simulink model "dark_pfb_core10"  (R2012b)
settings: Solver=ode45  StartTime=0.0  StopTime=10.0  RelTol=1e-3
undefined symbols (not defined in the file or in its folder): pfb_coeff_gen_calc
libraries: xbsIndex_r4 x32067, casper_library_misc x268, casper_library_delays x22, xps_library x1
(part 1 of 300)
settings Model: Creator=kids  LastModifiedBy=kids

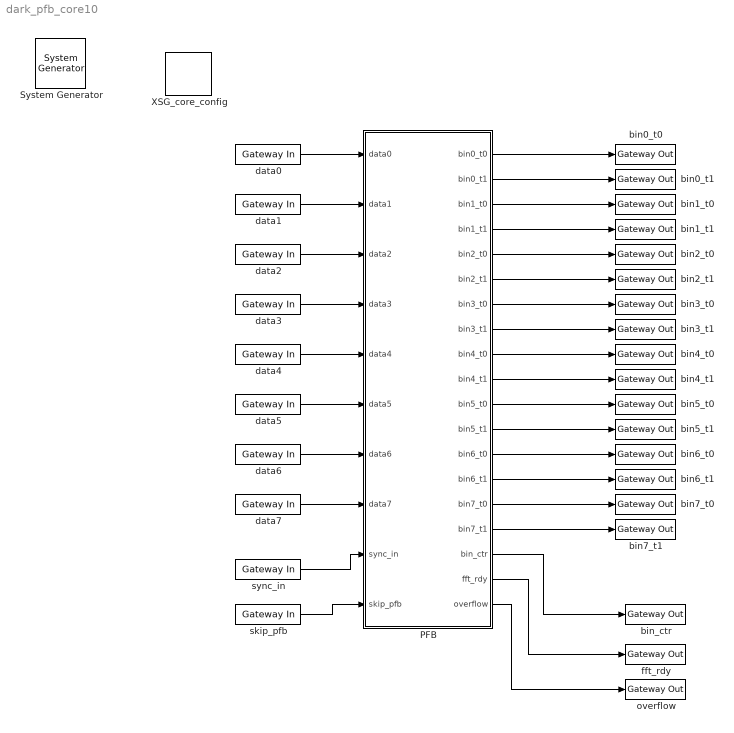
== system dark_pfb_core10 ==
  " System Generator" : xbsIndex_r4/ System Generator  Tag=genX  xilinxfamily=virtex6  part=xc6vsx475t  speed=-1  package=ff1759  synthesis_tool=XST  clock_wrapper=Clock Enables  directory=./dark_pfb_core10/sysgen  proj_type=Project Navigator  Synth_file=XST Defaults  Impl_file=ISE Defaults  testbench=off  simulink_period=1  sysclk_period=4  dcm_input_clock_period=100  incr_netlist=off  trim_vbits=Everywhere in SubSystem  dbl_ovrd=According to Block Masks  core_generation=According to Block Masks  run_coregen=off  deprecated_control=off  eval_field=0  block_type=sysgen
  PFB : Subsystem
  XSG_core_config : xps_library/XSG_core_config  Tag=xps:xsg  hw_sys=ROACH2:sx475t  clk_src=sys_clk  ROACH_clk_src=sys_clk  ROACH2_clk_src=sys_clk  MKDIG_clk_src=sys_clk  clk_rate=250  sample_period=1  synthesis_tool=XST
  bin0_t0 : xbsIndex_r4/Gateway Out  inherit_from_input=off  hdl_port=on  timing_constraint=None  locs_specified=off  LOCs={}  UseAsDAC=off  DACChannel='1'  block_type=gatewayout
  bin0_t1 : xbsIndex_r4/Gateway Out  inherit_from_input=off  hdl_port=on  timing_constraint=None  locs_specified=off  LOCs={}  UseAsDAC=off  DACChannel='1'  block_type=gatewayout
  bin1_t0 : xbsIndex_r4/Gateway Out  inherit_from_input=off  hdl_port=on  timing_constraint=None  locs_specified=off  LOCs={}  UseAsDAC=off  DACChannel='1'  block_type=gatewayout
  bin1_t1 : xbsIndex_r4/Gateway Out  inherit_from_input=off  hdl_port=on  timing_constraint=None  locs_specified=off  LOCs={}  UseAsDAC=off  DACChannel='1'  block_type=gatewayout
  bin2_t0 : xbsIndex_r4/Gateway Out  inherit_from_input=off  hdl_port=on  timing_constraint=None  locs_specified=off  LOCs={}  UseAsDAC=off  DACChannel='1'  block_type=gatewayout
  bin2_t1 : xbsIndex_r4/Gateway Out  inherit_from_input=off  hdl_port=on  timing_constraint=None  locs_specified=off  LOCs={}  UseAsDAC=off  DACChannel='1'  block_type=gatewayout
  bin3_t0 : xbsIndex_r4/Gateway Out  inherit_from_input=off  hdl_port=on  timing_constraint=None  locs_specified=off  LOCs={}  UseAsDAC=off  DACChannel='1'  block_type=gatewayout
  bin3_t1 : xbsIndex_r4/Gateway Out  inherit_from_input=off  hdl_port=on  timing_constraint=None  locs_specified=off  LOCs={}  UseAsDAC=off  DACChannel='1'  block_type=gatewayout
  bin4_t0 : xbsIndex_r4/Gateway Out  inherit_from_input=off  hdl_port=on  timing_constraint=None  locs_specified=off  LOCs={}  UseAsDAC=off  DACChannel='1'  block_type=gatewayout
  bin4_t1 : xbsIndex_r4/Gateway Out  inherit_from_input=off  hdl_port=on  timing_constraint=None  locs_specified=off  LOCs={}  UseAsDAC=off  DACChannel='1'  block_type=gatewayout
  bin5_t0 : xbsIndex_r4/Gateway Out  inherit_from_input=off  hdl_port=on  timing_constraint=None  locs_specified=off  LOCs={}  UseAsDAC=off  DACChannel='1'  block_type=gatewayout
  bin5_t1 : xbsIndex_r4/Gateway Out  inherit_from_input=off  hdl_port=on  timing_constraint=None  locs_specified=off  LOCs={}  UseAsDAC=off  DACChannel='1'  block_type=gatewayout
  bin6_t0 : xbsIndex_r4/Gateway Out  inherit_from_input=off  hdl_port=on  timing_constraint=None  locs_specified=off  LOCs={}  UseAsDAC=off  DACChannel='1'  block_type=gatewayout
  bin6_t1 : xbsIndex_r4/Gateway Out  inherit_from_input=off  hdl_port=on  timing_constraint=None  locs_specified=off  LOCs={}  UseAsDAC=off  DACChannel='1'  block_type=gatewayout
  bin7_t0 : xbsIndex_r4/Gateway Out  inherit_from_input=off  hdl_port=on  timing_constraint=None  locs_specified=off  LOCs={}  UseAsDAC=off  DACChannel='1'  block_type=gatewayout
  bin7_t1 : xbsIndex_r4/Gateway Out  inherit_from_input=off  hdl_port=on  timing_constraint=None  locs_specified=off  LOCs={}  UseAsDAC=off  DACChannel='1'  block_type=gatewayout
  bin_ctr : xbsIndex_r4/Gateway Out  inherit_from_input=off  hdl_port=on  timing_constraint=None  locs_specified=off  LOCs={}  UseAsDAC=off  DACChannel='1'  block_type=gatewayout
  data{0..7} (8 alike) : xbsIndex_r4/Gateway In  gui_display_data_type=Fixed-point  arith_type=Unsigned  n_bits=24  bin_pt=0  preci_type=Single  exp_width=8  frac_width=24  quantization="Round  (unbiased: +/- Inf)"  overflow=Saturate  period=1  dbl_ovrd=off  timing_constraint=None  locs_specified=off  LOCs={}  UseAsADC=off  ADCChannel='1'  inherit_from_input=off  hdl_port=on  block_type=gatewayin
  fft_rdy : xbsIndex_r4/Gateway Out  inherit_from_input=off  hdl_port=on  timing_constraint=None  locs_specified=off  LOCs={}  UseAsDAC=off  DACChannel='1'  block_type=gatewayout
  overflow : xbsIndex_r4/Gateway Out  inherit_from_input=off  hdl_port=on  timing_constraint=None  locs_specified=off  LOCs={}  UseAsDAC=off  DACChannel='1'  block_type=gatewayout
  skip_pfb : xbsIndex_r4/Gateway In  gui_display_data_type=Boolean  arith_type=Boolean  n_bits=16  bin_pt=14  preci_type=Single  exp_width=8  frac_width=24  quantization="Round  (unbiased: +/- Inf)"  overflow=Saturate  period=1  dbl_ovrd=off  timing_constraint=None  locs_specified=off  LOCs={}  UseAsADC=off  ADCChannel='1'  inherit_from_input=off  hdl_port=on  block_type=gatewayin
  sync_in : xbsIndex_r4/Gateway In  gui_display_data_type=Boolean  arith_type=Boolean  n_bits=16  bin_pt=14  preci_type=Single  exp_width=8  frac_width=24  quantization="Round  (unbiased: +/- Inf)"  overflow=Saturate  period=1  dbl_ovrd=off  timing_constraint=None  locs_specified=off  LOCs={}  UseAsADC=off  ADCChannel='1'  inherit_from_input=off  hdl_port=on  block_type=gatewayin
  wires:
  PFB/18(fft_rdy) -> fft_rdy/1
  data0/1 -> PFB/1(data0)
  data1/1 -> PFB/2(data1)
  data2/1 -> PFB/3(data2)
  data3/1 -> PFB/4(data3)
  data4/1 -> PFB/5(data4)
  data5/1 -> PFB/6(data5)
  data6/1 -> PFB/7(data6)
  data7/1 -> PFB/8(data7)
  sync_in/1 -> PFB/9(sync_in)
  PFB/19(overflow) -> overflow/1
  PFB/17(bin_ctr) -> bin_ctr/1
  skip_pfb/1 -> PFB/10(skip_pfb)
  PFB/16(bin7_t1) -> bin7_t1/1
  PFB/15(bin7_t0) -> bin7_t0/1
  PFB/14(bin6_t1) -> bin6_t1/1
  PFB/13(bin6_t0) -> bin6_t0/1
  PFB/12(bin5_t1) -> bin5_t1/1
  PFB/11(bin5_t0) -> bin5_t0/1
  PFB/10(bin4_t1) -> bin4_t1/1
  PFB/9(bin4_t0) -> bin4_t0/1
  PFB/8(bin3_t1) -> bin3_t1/1
  PFB/7(bin3_t0) -> bin3_t0/1
  PFB/6(bin2_t1) -> bin2_t1/1
  PFB/5(bin2_t0) -> bin2_t0/1
  PFB/4(bin1_t1) -> bin1_t1/1
  PFB/3(bin1_t0) -> bin1_t0/1
  PFB/2(bin0_t1) -> bin0_t1/1
  PFB/1(bin0_t0) -> bin0_t0/1

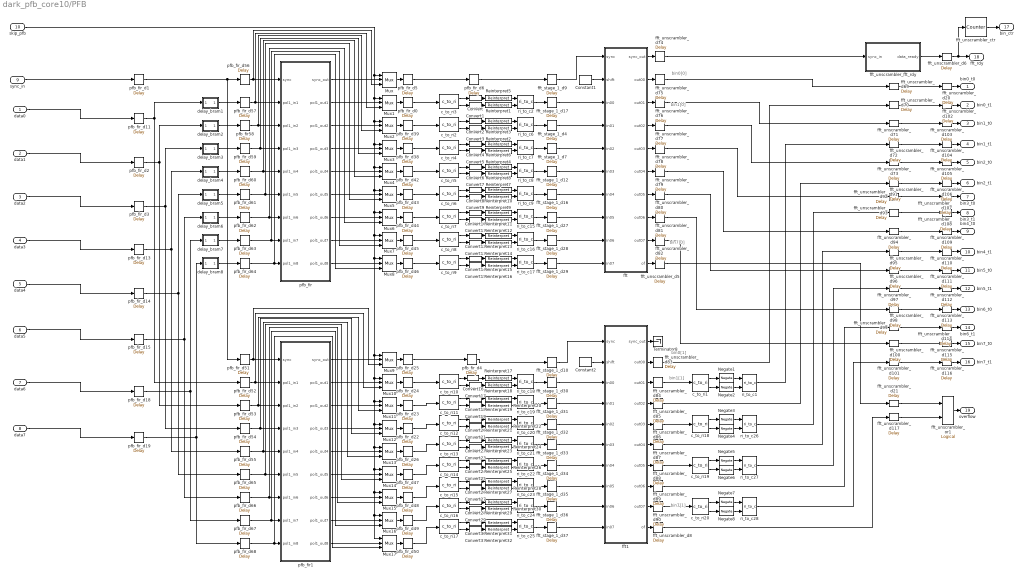
== system dark_pfb_core10/PFB ==
  note: bin7[1]
  note: bin7[0]
  note: bin1[1]
  note: bin1[0]
  note: bin0[1]
  note: bin0[0]
  data0 : Inport
  data1 : Inport  Port=2
  data2 : Inport  Port=3
  data3 : Inport  Port=4
  data4 : Inport  Port=5
  data5 : Inport  Port=6
  data6 : Inport  Port=7
  data7 : Inport  Port=8
  sync_in : Inport  Port=9
  skip_pfb : Inport  Port=10
  Constant1 : xbsIndex_r4/Constant  const=2^5-1  gui_display_data_type=Fixed-point  arith_type=Unsigned  n_bits=32  bin_pt=0  preci_type=Single  exp_width=8  frac_width=24  explicit_period=on  period=1  equ=P=C  opselect=C  inp2=PCIN>>17  opr=+  inp1=P  carry=CIN  dbl_ovrd=off  block_type=constant
  Constant2 : xbsIndex_r4/Constant  const=2^5-1  gui_display_data_type=Fixed-point  arith_type=Unsigned  n_bits=32  bin_pt=0  preci_type=Single  exp_width=8  frac_width=24  explicit_period=on  period=1  equ=P=C  opselect=C  inp2=PCIN>>17  opr=+  inp1=P  carry=CIN  dbl_ovrd=off  block_type=constant
  Convert{,1..31} (32 alike) : xbsIndex_r4/Convert  gui_display_data_type=Fixed-point  arith_type=Unsigned  n_bits=18  bin_pt=17  float_type=Single  exp_bits=8  fraction_bits=24  quantization="Round  (unbiased: +/- Inf)"  overflow=Wrap  en=off  latency=2  dbl_ovrd=off  pipeline=on  block_type=convert
      settings: block_version=11.4
  Mux{,1..17} (18 alike) : xbsIndex_r4/Mux  inputs=2  en=off  latency=0  precision=Full  arith_type=Unsigned  n_bits=16  bin_pt=14  quantization=Truncate  overflow=Wrap  dbl_ovrd=off  block_type=mux
  Negate{1..8} (8 alike) : xbsIndex_r4/Negate  precision=User Defined  arith_type="Signed  (2's comp)"  n_bits=18  bin_pt=17  quantization=Truncate  overflow=Wrap  en=off  latency=0  dbl_ovrd=off  block_type=negate
      settings: block_version=11.4
  Reinterpret{1..32} (32 alike) : xbsIndex_r4/Reinterpret  force_arith_type=off  arith_type="Signed  (2's comp)"  force_bin_pt=on  bin_pt=0  block_type=reinterpret
      settings: infoedit="Changes signal type without altering the binary representation.   You can changed the signal between signed and unsigned, and relocate the binary point.<br><br>Hardware notes: In hardware this block costs nothing.<br><br>Example:  Suppose the input is 6 bits wide, signed, with 2 fractional bits, and the output is forced to unsigned with 0 fractional bits.  Then an input of -2.0 (1110.00 in binary 2's complement) becomes an output of 56 (111000 in binary)."
  Terminator8 : Terminator
  c_to_ri{1,18..20} (4 alike) : casper_library_misc/c_to_ri  n_bits=18  bin_pt=17
  c_to_ri{2..17} (16 alike) : casper_library_misc/c_to_ri  n_bits=12  bin_pt=11
      settings: AttributesFormatString=12_11 r/i
  delay_bram1 : Subsystem  AncestorBlock=casper_library_delays/delay_bram  mask(delay_bram){DelayLen=128, bram_latency=2, use_dsp48=on, async=off}
      mask prompts: DelayLen "Delay By:"; bram_latency "BRAM Latency"; use_dsp48 "Use DSP48"; async "asynchronous operation"
      mask help: A delay block that uses BRAM for its storage.
      mask init:
      | delay_bram_init(gcb, ...
      |     'DelayLen', DelayLen, ...
      |     'bram_latency', bram_latency, ...
      |     'use_dsp48', use_dsp48, ...
      |     'async', async);
      mask Help: eval('xlWeb(''http://casper.berkeley.edu/wiki/Delay_bram'')')
      mask Display:
      | str=sprintf('z^{-%d}',DelayLen);
      | color('black');disp(str,'texmode','on');
  delay_bram2 : Subsystem  AncestorBlock=casper_library_delays/delay_bram  mask(delay_bram){DelayLen=128, bram_latency=2, use_dsp48=on, async=off}
      mask prompts: DelayLen "Delay By:"; bram_latency "BRAM Latency"; use_dsp48 "Use DSP48"; async "asynchronous operation"
      mask help: A delay block that uses BRAM for its storage.
      mask init:
      | delay_bram_init(gcb, ...
      |     'DelayLen', DelayLen, ...
      |     'bram_latency', bram_latency, ...
      |     'use_dsp48', use_dsp48, ...
      |     'async', async);
      mask Help: eval('xlWeb(''http://casper.berkeley.edu/wiki/Delay_bram'')')
      mask Display:
      | str=sprintf('z^{-%d}',DelayLen);
      | color('black');disp(str,'texmode','on');
  delay_bram3 : Subsystem  AncestorBlock=casper_library_delays/delay_bram  mask(delay_bram){DelayLen=128, bram_latency=2, use_dsp48=on, async=off}
      mask prompts: DelayLen "Delay By:"; bram_latency "BRAM Latency"; use_dsp48 "Use DSP48"; async "asynchronous operation"
      mask help: A delay block that uses BRAM for its storage.
      mask init:
      | delay_bram_init(gcb, ...
      |     'DelayLen', DelayLen, ...
      |     'bram_latency', bram_latency, ...
      |     'use_dsp48', use_dsp48, ...
      |     'async', async);
      mask Help: eval('xlWeb(''http://casper.berkeley.edu/wiki/Delay_bram'')')
      mask Display:
      | str=sprintf('z^{-%d}',DelayLen);
      | color('black');disp(str,'texmode','on');
  delay_bram4 : Subsystem  AncestorBlock=casper_library_delays/delay_bram  mask(delay_bram){DelayLen=128, bram_latency=2, use_dsp48=on, async=off}
      mask prompts: DelayLen "Delay By:"; bram_latency "BRAM Latency"; use_dsp48 "Use DSP48"; async "asynchronous operation"
      mask help: A delay block that uses BRAM for its storage.
      mask init:
      | delay_bram_init(gcb, ...
      |     'DelayLen', DelayLen, ...
      |     'bram_latency', bram_latency, ...
      |     'use_dsp48', use_dsp48, ...
      |     'async', async);
      mask Help: eval('xlWeb(''http://casper.berkeley.edu/wiki/Delay_bram'')')
      mask Display:
      | str=sprintf('z^{-%d}',DelayLen);
      | color('black');disp(str,'texmode','on');
  delay_bram5 : Subsystem  AncestorBlock=casper_library_delays/delay_bram  mask(delay_bram){DelayLen=128, bram_latency=2, use_dsp48=on, async=off}
      mask prompts: DelayLen "Delay By:"; bram_latency "BRAM Latency"; use_dsp48 "Use DSP48"; async "asynchronous operation"
      mask help: A delay block that uses BRAM for its storage.
      mask init:
      | delay_bram_init(gcb, ...
      |     'DelayLen', DelayLen, ...
      |     'bram_latency', bram_latency, ...
      |     'use_dsp48', use_dsp48, ...
      |     'async', async);
      mask Help: eval('xlWeb(''http://casper.berkeley.edu/wiki/Delay_bram'')')
      mask Display:
      | str=sprintf('z^{-%d}',DelayLen);
      | color('black');disp(str,'texmode','on');
  delay_bram6 : Subsystem  AncestorBlock=casper_library_delays/delay_bram  mask(delay_bram){DelayLen=128, bram_latency=2, use_dsp48=on, async=off}
      mask prompts: DelayLen "Delay By:"; bram_latency "BRAM Latency"; use_dsp48 "Use DSP48"; async "asynchronous operation"
      mask help: A delay block that uses BRAM for its storage.
      mask init:
      | delay_bram_init(gcb, ...
      |     'DelayLen', DelayLen, ...
      |     'bram_latency', bram_latency, ...
      |     'use_dsp48', use_dsp48, ...
      |     'async', async);
      mask Help: eval('xlWeb(''http://casper.berkeley.edu/wiki/Delay_bram'')')
      mask Display:
      | str=sprintf('z^{-%d}',DelayLen);
      | color('black');disp(str,'texmode','on');
  delay_bram7 : Subsystem  AncestorBlock=casper_library_delays/delay_bram  mask(delay_bram){DelayLen=128, bram_latency=2, use_dsp48=on, async=off}
      mask prompts: DelayLen "Delay By:"; bram_latency "BRAM Latency"; use_dsp48 "Use DSP48"; async "asynchronous operation"
      mask help: A delay block that uses BRAM for its storage.
      mask init:
      | delay_bram_init(gcb, ...
      |     'DelayLen', DelayLen, ...
      |     'bram_latency', bram_latency, ...
      |     'use_dsp48', use_dsp48, ...
      |     'async', async);
      mask Help: eval('xlWeb(''http://casper.berkeley.edu/wiki/Delay_bram'')')
      mask Display:
      | str=sprintf('z^{-%d}',DelayLen);
      | color('black');disp(str,'texmode','on');
  delay_bram8 : Subsystem  AncestorBlock=casper_library_delays/delay_bram  mask(delay_bram){DelayLen=128, bram_latency=2, use_dsp48=on, async=off}
      mask prompts: DelayLen "Delay By:"; bram_latency "BRAM Latency"; use_dsp48 "Use DSP48"; async "asynchronous operation"
      mask help: A delay block that uses BRAM for its storage.
      mask init:
      | delay_bram_init(gcb, ...
      |     'DelayLen', DelayLen, ...
      |     'bram_latency', bram_latency, ...
      |     'use_dsp48', use_dsp48, ...
      |     'async', async);
      mask Help: eval('xlWeb(''http://casper.berkeley.edu/wiki/Delay_bram'')')
      mask Display:
      | str=sprintf('z^{-%d}',DelayLen);
      | color('black');disp(str,'texmode','on');
  fft : Subsystem  Tag=casper:fft  AncestorBlock=casper_library_ffts/fft  mask(fft){n_streams=1, FFTSize=11, n_inputs=3, input_bit_width=18, bin_pt_in=-1, coeff_bit_width=18, async=off, unscramble=on, add_latency=2, mult_latency=2, bram_latency=3, conv_latency=3, quantization=Truncate, overflow=Wrap, delays_bit_limit=8, coeffs_bit_limit=8, coeff_sharing=on, coeff_decimation=on, coeff_generation=on, cal_bits=1, n_bits_rotation=25, max_fanout=4, mult_spec=2, bitgrowth=off, max_bits=19, hardcode_shifts=off, shift_schedule=0, dsp48_adders=on}
      mask prompts: n_streams "Number input streams"; FFTSize "Size of FFT: (2^? pts)"; n_inputs "Number of Simultaneous Inputs: (2^?)"; input_bit_width "Input bit width"; bin_pt_in "Input binary point"; coeff_bit_width "Coefficient Bit Width"; async "Asynchronous operation"; unscramble "Unscramble output (ie, put channels in canonical order)"; add_latency "Add Latency"; mult_latency "Mult Latency"; bram_latency "BRAM Latency"; conv_latency "Convert Latency"; quantization "Quantization Behavior"; overflow "Overflow Behavior"; delays_bit_limit "Number bits above which to store stage's delays in BRAM (2^? bits)"; coeffs_bit_limit "Number bits above which to store stage's coefficients in BRAM (2^? bits)"; coeff_sharing "BRAM sharing in coeff storage"; coeff_decimation "Store a fraction of coeff factors where useful"; coeff_generation "Generate coeffs with multipliers where useful"; cal_bits "Number calibration locations when generating coeffs (2^?)"; n_bits_rotation "Feedback rotation vector resolution"; max_fanout "Maximum fanout"; mult_spec "Multiplier impl. (0=core, 1=embedded, 2=behavioural) (left=1st stage, or just one value)"; bitgrowth "Bitgrowth instead of shifting"; max_bits "Max bits to grow to"; hardcode_shifts "Hardcode shift schedule"; shift_schedule "Shift schedule"; dsp48_adders "DSP48 adders in butterfly"
      mask help: A complex FFT.
      mask init:
      | fft_init(gcb, ...
      |     'n_streams', n_streams, ...
      |     'FFTSize', FFTSize, ...
      |     'n_inputs', n_inputs, ...
      |     'input_bit_width', input_bit_width, ...
      |     'coeff_bit_width', coeff_bit_width, ...
      |     'async', async, ...
      |     'unscramble', unscramble, ...
      |     'add_latency', add_latency, ...
      |     'mult_latency', mult_latency, ...
      |     'bram_latency', bram_latency, ...
      |     'conv_latency', conv_latency, ...
      |     'quantization', quantization, ...
      |     'overflow', overflow, ...
      |     'delays_bit_limit', delays_bit_limit, ...
      |     'coeffs_bit_limit', coeffs_bit_limit, ...
      |     'coeff_sharing', coeff_sharing, ...
      |     'coeff_decimation', coeff_decimation, ...
      |     'coeff_generation', coeff_generation, ...
      |     'cal_bits', cal_bits, ...
      |     'n_bits_rotation', n_bits_rotation, ...
      |     'max_fanout', max_fanout, ...   
      |     'mult_spec', mult_spec, ...
      |     'bitgrowth', bitgrowth, ...
      |     'hardcode_shifts', hardcode_shifts, ...
      |     'shift_schedule', shift_schedule, ...
      |     'dsp48_adders', dsp48_adders);
      settings: AttributesFormatString=|
      | 11 stages
      | (18,18)
      | Truncate
      | Wrap
      mask Help: eval('xlWeb(''http://casper.berkeley.edu/wiki/Fft'')')
      mask TypeOptions quantization: Truncate | Round  (unbiased: +/- Inf) | Round  (unbiased: Even Values)
      mask TypeOptions overflow: Wrap | Saturate | Error
      mask Callback hardcode_shifts:
      | hardcode_shifts = get_param(gcb, 'hardcode_shifts');
      | set_param_state(gcb, 'shift_schedule', hardcode_shifts);
      mask Callback dsp48_adders: fft_callback_dsp48_adders(gcb);
  fft1 : Subsystem  Tag=casper:fft  AncestorBlock=casper_library_ffts/fft  mask(fft){n_streams=1, FFTSize=11, n_inputs=3, input_bit_width=18, bin_pt_in=-1, coeff_bit_width=18, async=off, unscramble=on, add_latency=2, mult_latency=2, bram_latency=3, conv_latency=3, quantization=Truncate, overflow=Wrap, delays_bit_limit=8, coeffs_bit_limit=8, coeff_sharing=on, coeff_decimation=on, coeff_generation=on, cal_bits=1, n_bits_rotation=25, max_fanout=4, mult_spec=2, bitgrowth=off, max_bits=19, hardcode_shifts=off, shift_schedule=0, dsp48_adders=on}
      mask prompts: n_streams "Number input streams"; FFTSize "Size of FFT: (2^? pts)"; n_inputs "Number of Simultaneous Inputs: (2^?)"; input_bit_width "Input bit width"; bin_pt_in "Input binary point"; coeff_bit_width "Coefficient Bit Width"; async "Asynchronous operation"; unscramble "Unscramble output (ie, put channels in canonical order)"; add_latency "Add Latency"; mult_latency "Mult Latency"; bram_latency "BRAM Latency"; conv_latency "Convert Latency"; quantization "Quantization Behavior"; overflow "Overflow Behavior"; delays_bit_limit "Number bits above which to store stage's delays in BRAM (2^? bits)"; coeffs_bit_limit "Number bits above which to store stage's coefficients in BRAM (2^? bits)"; coeff_sharing "BRAM sharing in coeff storage"; coeff_decimation "Store a fraction of coeff factors where useful"; coeff_generation "Generate coeffs with multipliers where useful"; cal_bits "Number calibration locations when generating coeffs (2^?)"; n_bits_rotation "Feedback rotation vector resolution"; max_fanout "Maximum fanout"; mult_spec "Multiplier impl. (0=core, 1=embedded, 2=behavioural) (left=1st stage, or just one value)"; bitgrowth "Bitgrowth instead of shifting"; max_bits "Max bits to grow to"; hardcode_shifts "Hardcode shift schedule"; shift_schedule "Shift schedule"; dsp48_adders "DSP48 adders in butterfly"
      mask help: A complex FFT.
      mask init: same as dark_pfb_core10/PFB/fft
      settings: AttributesFormatString=|
      | 11 stages
      | (18,18)
      | Truncate
      | Wrap
      mask Help: eval('xlWeb(''http://casper.berkeley.edu/wiki/Fft'')')
      mask TypeOptions quantization: Truncate | Round  (unbiased: +/- Inf) | Round  (unbiased: Even Values)
      mask TypeOptions overflow: Wrap | Saturate | Error
      mask Callback hardcode_shifts:
      | hardcode_shifts = get_param(gcb, 'hardcode_shifts');
      | set_param_state(gcb, 'shift_schedule', hardcode_shifts);
      mask Callback dsp48_adders: fft_callback_dsp48_adders(gcb);
  fft_stage_1_d{4,7,9..10,12,16..17,27..37} (18 alike) : xbsIndex_r4/Delay  rst=off  infoeditControl=Selection of Reset will increase slice count due to use of real FFs and instead of SRLs  en=off  latency=2  dbl_ovrd=off  reg_retiming=off  block_type=delay
      settings: xl_area=[18 36 0 0 0 0 0]
  fft_unscrambler_ctr : xbsIndex_r4/Counter  cnt_type=Free Running  cnt_to=Inf  operation=Up  start_count=0  cnt_by_val=1  arith_type=Unsigned  n_bits=8  bin_pt=0  load_pin=off  rst=on  en=off  explicit_period=on  period=1  dbl_ovrd=off  use_behavioral_HDL=off  implementation=Fabric  block_type=counter
  fft_unscrambler_d{21,69..73,91..101,117} (18 alike) : xbsIndex_r4/Delay  rst=off  infoeditControl=Selection of Reset will increase slice count due to use of real FFs and instead of SRLs  en=off  latency=2  dbl_ovrd=off  reg_retiming=off  block_type=delay
      settings: xl_area=[18 36 0 0 0 0 0]
  fft_unscrambler_d{5..6,8,20,74..90,102..116} (36 alike) : xbsIndex_r4/Delay  rst=off  infoeditControl=Selection of Reset will increase slice count due to use of real FFs and instead of SRLs  en=off  latency=1  dbl_ovrd=off  reg_retiming=off  block_type=delay
      settings: xl_area=[18 36 0 0 0 0 0]
  fft_unscrambler_fft_rdy : Subsystem
  fft_unscrambler_or1 : xbsIndex_r4/Logical  logical_function=OR  inputs=2  en=off  latency=1  precision=Full  arith_type=Unsigned  n_bits=16  bin_pt=0  align_bp=on  dbl_ovrd=off  block_type=logical
  pfb_fir : Subsystem  Tag=casper:pfb_fir  AncestorBlock=casper_library_pfbs/pfb_fir  mask(pfb_fir){PFBSize=11, TotalTaps=4, WindowType=hamming, n_inputs=3, MakeBiplex=off, BitWidthIn=12, BitWidthOut=12, CoeffBitWidth=12, CoeffDistMem=off, add_latency=1, mult_latency=2, bram_latency=3, fan_latency=1, quantization=Truncate, fwidth=2., mult_spec=2, coeffs_share=on}
      mask prompts: PFBSize "Size of PFB: (2^? pnts)"; TotalTaps "Total Number of Taps:"; WindowType "Windowing Function:"; n_inputs "Number of Simultaneous Inputs: (2^?)"; MakeBiplex "Make Biplex?"; BitWidthIn "Input Bitwidth:"; BitWidthOut "Output Bitwidth:"; CoeffBitWidth "Coefficient Bitwidth:"; CoeffDistMem "Use Distributed Memory for Coeffs"; add_latency "Add Latency"; mult_latency "Mult Latency"; bram_latency "BRAM Latency"; fan_latency "Fanout Latency"; quantization "Quantization Behavior"; fwidth "Bin Width Scaling (normal=1)"; mult_spec "Multiplier specification (0=core, 1=embedded, 2=behavioural) (left=1st tap, or just one value)"; coeffs_share "Share coefficients between polarisations (use less BRAM)?"
      mask init:
      | pfb_fir_init(gcb, ...
      |     'PFBSize', PFBSize, ...
      |     'TotalTaps', TotalTaps, ...
      |     'WindowType', WindowType, ...
      |     'n_inputs', n_inputs, ...
      |     'MakeBiplex', MakeBiplex, ...
      |     'BitWidthIn', BitWidthIn, ...
      |     'BitWidthOut', BitWidthOut, ...
      |     'CoeffBitWidth', CoeffBitWidth, ...
      |     'CoeffDistMem', CoeffDistMem, ...
      |     'add_latency', add_latency, ...
      |     'mult_latency', mult_latency, ...
      |     'bram_latency', bram_latency, ...
      |     'fan_latency', fan_latency, ...
      |     'quantization', quantization, ...
      |     'fwidth', fwidth, ...
      |     'mult_spec', mult_spec, ...
      |     'coeffs_share', coeffs_share);
      settings: AttributesFormatString=|
      | taps=4, add_latency=1
      | max scale 0.521
      mask Help: eval('xlWeb(''http://casper.berkeley.edu/wiki/Pfb_fir'')')
      mask Callback TotalTaps:
      | %num_taps = eval(get_param(gcb, 'TotalTaps'));
      | %old_vec = eval(get_param(gcb, 'mult_spec'));
      | 
      | %vec = 2.*ones(1,num_taps);
      | %old_size = length(old_vec);
      | 
      | %if(old_size <= num_taps),
      | %   new_size = old_size;
      | %else,
      | %    new_size = num_taps;
      | %end
      | 
      | %for n = 1:new_size, vec(n) = old_vec(n); end
      | %set_param(gcb, 'mult_spec', mat2str(vec));
      mask TypeOptions WindowType: bartlett | barthannwin | blackman | blackmanharris | bohamwin | chebwin | flattopwin | gausswin | hamming | hann | kaiser | nuttallwin | parzenwin | rectwin | tukeywin | triang | userwindow
      mask TypeOptions quantization: Truncate | Round  (unbiased: +/- Inf) | Round  (unbiased: Even Values)
      mask Callback fwidth:
      | %num_taps = eval(get_param(gcb, 'TotalTaps'));
      | %old_vec = eval(get_param(gcb, 'mult_spec'));
      | 
      | %vec = 2.*ones(1,num_taps);
      | %old_size = length(old_vec);
      | 
      | %if(old_size <= num_taps),
      | %    new_size = old_size;
      | %else,
      | %    new_size = num_taps;
      | %end
      | 
      | %for n = 1:new_size, vec(n) = old_vec(n); end
      | %set_param(gcb, 'mult_spec', mat2str(vec));
      mask Callback mult_spec:
      | %num_taps = eval(get_param(gcb, 'TotalTaps'));
      | %old_vec = eval(get_param(gcb, 'mult_spec'));
      | 
      | %vec = 2.*ones(1,num_taps);
      | %old_size = length(old_vec);
      | 
      | %if(old_size <= num_taps),
      | %    new_size = old_size;
      | %else,
      | %    new_size = num_taps;
      | %end
      | 
      | %for n = 1:new_size, vec(n) = old_vec(n); end
      | %set_param(gcb, 'mult_spec', mat2str(vec));
  pfb_fir1 : Subsystem  Tag=casper:pfb_fir  AncestorBlock=casper_library_pfbs/pfb_fir  mask(pfb_fir){PFBSize=11, TotalTaps=4, WindowType=hamming, n_inputs=3, MakeBiplex=off, BitWidthIn=12, BitWidthOut=12, CoeffBitWidth=12, CoeffDistMem=off, add_latency=1, mult_latency=2, bram_latency=3, fan_latency=1, quantization=Truncate, fwidth=2., mult_spec=2, coeffs_share=on}
      mask prompts: PFBSize "Size of PFB: (2^? pnts)"; TotalTaps "Total Number of Taps:"; WindowType "Windowing Function:"; n_inputs "Number of Simultaneous Inputs: (2^?)"; MakeBiplex "Make Biplex?"; BitWidthIn "Input Bitwidth:"; BitWidthOut "Output Bitwidth:"; CoeffBitWidth "Coefficient Bitwidth:"; CoeffDistMem "Use Distributed Memory for Coeffs"; add_latency "Add Latency"; mult_latency "Mult Latency"; bram_latency "BRAM Latency"; fan_latency "Fanout Latency"; quantization "Quantization Behavior"; fwidth "Bin Width Scaling (normal=1)"; mult_spec "Multiplier specification (0=core, 1=embedded, 2=behavioural) (left=1st tap, or just one value)"; coeffs_share "Share coefficients between polarisations (use less BRAM)?"
      mask init: same as dark_pfb_core10/PFB/pfb_fir
      settings: AttributesFormatString=|
      | taps=4, add_latency=1
      | max scale 0.521
      mask Help: eval('xlWeb(''http://casper.berkeley.edu/wiki/Pfb_fir'')')
      mask Callback TotalTaps:
      | %num_taps = eval(get_param(gcb, 'TotalTaps'));
      | %old_vec = eval(get_param(gcb, 'mult_spec'));
      | 
      | %vec = 2.*ones(1,num_taps);
      | %old_size = length(old_vec);
      | 
      | %if(old_size <= num_taps),
      | %   new_size = old_size;
      | %else,
      | %    new_size = num_taps;
      | %end
      | 
      | %for n = 1:new_size, vec(n) = old_vec(n); end
      | %set_param(gcb, 'mult_spec', mat2str(vec));
      mask TypeOptions WindowType: bartlett | barthannwin | blackman | blackmanharris | bohamwin | chebwin | flattopwin | gausswin | hamming | hann | kaiser | nuttallwin | parzenwin | rectwin | tukeywin | triang | userwindow
      mask TypeOptions quantization: Truncate | Round  (unbiased: +/- Inf) | Round  (unbiased: Even Values)
      mask Callback fwidth:
      | %num_taps = eval(get_param(gcb, 'TotalTaps'));
      | %old_vec = eval(get_param(gcb, 'mult_spec'));
      | 
      | %vec = 2.*ones(1,num_taps);
      | %old_size = length(old_vec);
      | 
      | %if(old_size <= num_taps),
      | %    new_size = old_size;
      | %else,
      | %    new_size = num_taps;
      | %end
      | 
      | %for n = 1:new_size, vec(n) = old_vec(n); end
      | %set_param(gcb, 'mult_spec', mat2str(vec));
      mask Callback mult_spec:
      | %num_taps = eval(get_param(gcb, 'TotalTaps'));
      | %old_vec = eval(get_param(gcb, 'mult_spec'));
      | 
      | %vec = 2.*ones(1,num_taps);
      | %old_size = length(old_vec);
      | 
      | %if(old_size <= num_taps),
      | %    new_size = old_size;
      | %else,
      | %    new_size = num_taps;
      | %end
      | 
      | %for n = 1:new_size, vec(n) = old_vec(n); end
      | %set_param(gcb, 'mult_spec', mat2str(vec));
  pfb_fir58 : xbsIndex_r4/Delay  rst=off  infoeditControl=Selection of Reset will increase slice count due to use of real FFs and instead of SRLs  en=off  latency=2  dbl_ovrd=off  reg_retiming=off  block_type=delay
      settings: xl_area=[18 36 0 0 0 0 0]
  pfb_fir_d{0,4..6,22..26,38..39,42..57,59..68} (37 alike) : xbsIndex_r4/Delay  rst=off  infoeditControl=Selection of Reset will increase slice count due to use of real FFs and instead of SRLs  en=off  latency=2  dbl_ovrd=off  reg_retiming=off  block_type=delay
      settings: xl_area=[18 36 0 0 0 0 0]
  pfb_fir_d{1..3,11,13..15,18..19} (9 alike) : xbsIndex_r4/Delay  rst=off  infoeditControl=Selection of Reset will increase slice count due to use of real FFs and instead of SRLs  en=off  latency=1  dbl_ovrd=off  reg_retiming=off  block_type=delay
      settings: xl_area=[18 36 0 0 0 0 0]
  ri_to_c{1..2,6..9,15..28} (20 alike) : casper_library_misc/ri_to_c
  bin0_t0 : Outport
  bin0_t1 : Outport  Port=2
  bin1_t0 : Outport  Port=3
  bin1_t1 : Outport  Port=4
  bin2_t0 : Outport  Port=5
  bin2_t1 : Outport  Port=6
  bin3_t0 : Outport  Port=7
  bin3_t1 : Outport  Port=8
  bin4_t0 : Outport  Port=9
  bin4_t1 : Outport  Port=10
  bin5_t0 : Outport  Port=11
  bin5_t1 : Outport  Port=12
  bin6_t0 : Outport  Port=13
  bin6_t1 : Outport  Port=14
  bin7_t0 : Outport  Port=15
  bin7_t1 : Outport  Port=16
  bin_ctr : Outport  Port=17
  fft_rdy : Outport  Port=18
  overflow : Outport  Port=19
  wires:
  sync_in/1 -> pfb_fir_d1/1
  data0/1 -> pfb_fir_d11/1
  fft/1(sync_out) -> fft_unscrambler_d74/1
  pfb_fir_d1/1 -> pfb_fir_d51/1, pfb_fir_d56/1
  pfb_fir_d11/1 -> pfb_fir_d52/1, delay_bram1/1(din)
  Constant1/1 -> fft/2(shift)
  fft_unscrambler_d87/1 -> fft_unscrambler_d95/1
  fft_unscrambler_fft_rdy/1(data_ready) -> fft_unscrambler_d6/1
  fft_unscrambler_d6/1 -> fft_unscrambler_ctr/1, fft_rdy/1
  data1/1 -> pfb_fir_d2/1
  pfb_fir_d2/1 -> pfb_fir_d53/1, delay_bram2/1(din)
  delay_bram1/1(dout) -> pfb_fir_d57/1
  delay_bram2/1(dout) -> pfb_fir58/1
  fft_stage_1_d9/1 -> fft/1(sync)
  fft_stage_1_d10/1 -> fft1/1(sync)
  fft_stage_1_d17/1 -> fft/3(in00)
  c_to_ri1/1 -> Negate1/1
  c_to_ri1/2 -> Negate2/1
  Negate1/1 -> ri_to_c1/1
  Negate2/1 -> ri_to_c1/2
  c_to_ri3/1 -> Convert/1
  c_to_ri3/2 -> Convert1/1
  Convert/1 -> Reinterpret5/1
  Convert1/1 -> Reinterpret1/1
  Reinterpret5/1 -> ri_to_c2/1
  Reinterpret1/1 -> ri_to_c2/2
  ri_to_c2/1 -> fft_stage_1_d17/1
  Constant2/1 -> fft1/2(shift)
  fft1/3(out01) -> fft_unscrambler_d84/1
  pfb_fir_d5/1 -> pfb_fir_d6/1
  pfb_fir_d0/1 -> c_to_ri3/1
  pfb_fir_d25/1 -> pfb_fir_d4/1
  fft1/1(sync_out) -> Terminator8/1
  data2/1 -> pfb_fir_d3/1
  data3/1 -> pfb_fir_d13/1
  data4/1 -> pfb_fir_d14/1
  data5/1 -> pfb_fir_d15/1
  data6/1 -> pfb_fir_d18/1
  data7/1 -> pfb_fir_d19/1
  pfb_fir_d3/1 -> pfb_fir_d54/1, delay_bram3/1(din)
  pfb_fir_d13/1 -> pfb_fir_d55/1, delay_bram4/1(din)
  delay_bram3/1(dout) -> pfb_fir_d59/1
  delay_bram4/1(dout) -> pfb_fir_d60/1
  delay_bram5/1(dout) -> pfb_fir_d61/1
  delay_bram6/1(dout) -> pfb_fir_d62/1
  delay_bram7/1(dout) -> pfb_fir_d63/1
  delay_bram8/1(dout) -> pfb_fir_d64/1
  pfb_fir_d14/1 -> pfb_fir_d65/1, delay_bram5/1(din)
  pfb_fir_d15/1 -> pfb_fir_d66/1, delay_bram6/1(din)
  pfb_fir_d18/1 -> pfb_fir_d67/1, delay_bram7/1(din)
  pfb_fir_d19/1 -> pfb_fir_d68/1, delay_bram8/1(din)
  c_to_ri2/1 -> Convert2/1
  c_to_ri2/2 -> Convert3/1
  Convert2/1 -> Reinterpret3/1
  Convert3/1 -> Reinterpret2/1
  Reinterpret3/1 -> ri_to_c6/1
  Reinterpret2/1 -> ri_to_c6/2
  ri_to_c6/1 -> fft_stage_1_d4/1
  pfb_fir_d39/1 -> c_to_ri2/1
  c_to_ri4/1 -> Convert4/1
  c_to_ri4/2 -> Convert5/1
  Convert4/1 -> Reinterpret6/1
  Convert5/1 -> Reinterpret4/1
  Reinterpret6/1 -> ri_to_c7/1
  Reinterpret4/1 -> ri_to_c7/2
  ri_to_c7/1 -> fft_stage_1_d7/1
  pfb_fir_d38/1 -> c_to_ri4/1
  c_to_ri5/1 -> Convert6/1
  c_to_ri5/2 -> Convert7/1
  Convert6/1 -> Reinterpret8/1
  Convert7/1 -> Reinterpret7/1
  Reinterpret8/1 -> ri_to_c8/1
  Reinterpret7/1 -> ri_to_c8/2
  ri_to_c8/1 -> fft_stage_1_d12/1
  pfb_fir_d42/1 -> c_to_ri5/1
  c_to_ri6/1 -> Convert8/1
  c_to_ri6/2 -> Convert9/1
  Convert8/1 -> Reinterpret10/1
  Convert9/1 -> Reinterpret9/1
  Reinterpret10/1 -> ri_to_c9/1
  Reinterpret9/1 -> ri_to_c9/2
  ri_to_c9/1 -> fft_stage_1_d16/1
  pfb_fir_d43/1 -> c_to_ri6/1
  c_to_ri7/1 -> Convert10/1
  c_to_ri7/2 -> Convert11/1
  Convert10/1 -> Reinterpret11/1
  Convert11/1 -> Reinterpret12/1
  Reinterpret11/1 -> ri_to_c15/1
  Reinterpret12/1 -> ri_to_c15/2
  ri_to_c15/1 -> fft_stage_1_d27/1
  pfb_fir_d44/1 -> c_to_ri7/1
  c_to_ri8/1 -> Convert12/1
  c_to_ri8/2 -> Convert13/1
  Convert12/1 -> Reinterpret13/1
  Convert13/1 -> Reinterpret14/1
  Reinterpret13/1 -> ri_to_c16/1
  Reinterpret14/1 -> ri_to_c16/2
  ri_to_c16/1 -> fft_stage_1_d28/1
  pfb_fir_d45/1 -> c_to_ri8/1
  c_to_ri9/1 -> Convert14/1
  c_to_ri9/2 -> Convert15/1
  Convert14/1 -> Reinterpret15/1
  Convert15/1 -> Reinterpret16/1
  Reinterpret15/1 -> ri_to_c17/1
  Reinterpret16/1 -> ri_to_c17/2
  ri_to_c17/1 -> fft_stage_1_d29/1
  pfb_fir_d46/1 -> c_to_ri9/1
  c_to_ri10/1 -> Convert16/1
  c_to_ri10/2 -> Convert17/1
  Convert16/1 -> Reinterpret17/1
  Convert17/1 -> Reinterpret18/1
  Reinterpret17/1 -> ri_to_c18/1
  Reinterpret18/1 -> ri_to_c18/2
  ri_to_c18/1 -> fft_stage_1_d30/1
  pfb_fir_d24/1 -> c_to_ri10/1
  c_to_ri11/1 -> Convert18/1
  c_to_ri11/2 -> Convert19/1
  Convert18/1 -> Reinterpret19/1
  Convert19/1 -> Reinterpret20/1
  Reinterpret19/1 -> ri_to_c19/1
  Reinterpret20/1 -> ri_to_c19/2
  ri_to_c19/1 -> fft_stage_1_d31/1
  pfb_fir_d23/1 -> c_to_ri11/1
  c_to_ri12/1 -> Convert20/1
  c_to_ri12/2 -> Convert21/1
  Convert20/1 -> Reinterpret21/1
  Convert21/1 -> Reinterpret22/1
  Reinterpret21/1 -> ri_to_c20/1
  Reinterpret22/1 -> ri_to_c20/2
  ri_to_c20/1 -> fft_stage_1_d32/1
  pfb_fir_d22/1 -> c_to_ri12/1
  c_to_ri13/1 -> Convert22/1
  c_to_ri13/2 -> Convert23/1
  Convert22/1 -> Reinterpret23/1
  Convert23/1 -> Reinterpret24/1
  Reinterpret23/1 -> ri_to_c21/1
  Reinterpret24/1 -> ri_to_c21/2
  ri_to_c21/1 -> fft_stage_1_d33/1
  pfb_fir_d26/1 -> c_to_ri13/1
  c_to_ri14/1 -> Convert24/1
  c_to_ri14/2 -> Convert25/1
  Convert24/1 -> Reinterpret25/1
  Convert25/1 -> Reinterpret26/1
  Reinterpret25/1 -> ri_to_c22/1
  Reinterpret26/1 -> ri_to_c22/2
  ri_to_c22/1 -> fft_stage_1_d34/1
  pfb_fir_d47/1 -> c_to_ri14/1
  c_to_ri15/1 -> Convert26/1
  c_to_ri15/2 -> Convert27/1
  Convert26/1 -> Reinterpret27/1
  Convert27/1 -> Reinterpret28/1
  Reinterpret27/1 -> ri_to_c23/1
  Reinterpret28/1 -> ri_to_c23/2
  ri_to_c23/1 -> fft_stage_1_d35/1
  pfb_fir_d48/1 -> c_to_ri15/1
  c_to_ri16/1 -> Convert28/1
  c_to_ri16/2 -> Convert29/1
  Convert28/1 -> Reinterpret29/1
  Convert29/1 -> Reinterpret30/1
  Reinterpret29/1 -> ri_to_c24/1
  Reinterpret30/1 -> ri_to_c24/2
  ri_to_c24/1 -> fft_stage_1_d36/1
  pfb_fir_d49/1 -> c_to_ri16/1
  c_to_ri17/1 -> Convert30/1
  c_to_ri17/2 -> Convert31/1
  Convert30/1 -> Reinterpret31/1
  Convert31/1 -> Reinterpret32/1
  Reinterpret31/1 -> ri_to_c25/1
  Reinterpret32/1 -> ri_to_c25/2
  ri_to_c25/1 -> fft_stage_1_d37/1
  pfb_fir_d50/1 -> c_to_ri17/1
  fft_stage_1_d30/1 -> fft1/3(in00)
  fft_stage_1_d31/1 -> fft1/4(in01)
  fft_stage_1_d32/1 -> fft1/5(in02)
  fft_stage_1_d33/1 -> fft1/6(in03)
  fft_stage_1_d34/1 -> fft1/7(in04)
  fft_stage_1_d35/1 -> fft1/8(in05)
  fft_stage_1_d36/1 -> fft1/9(in06)
  fft_stage_1_d37/1 -> fft1/10(in07)
  fft_stage_1_d4/1 -> fft/4(in01)
  fft_stage_1_d7/1 -> fft/5(in02)
  fft_stage_1_d12/1 -> fft/6(in03)
  fft_stage_1_d16/1 -> fft/7(in04)
  fft_stage_1_d27/1 -> fft/8(in05)
  fft_stage_1_d28/1 -> fft/9(in06)
  fft_stage_1_d29/1 -> fft/10(in07)
  fft1/10(of) -> fft_unscrambler_d8/1
  fft/10(of) -> fft_unscrambler_d5/1
  c_to_ri18/1 -> Negate3/1
  Negate3/1 -> ri_to_c26/1
  fft1/5(out03) -> fft_unscrambler_d86/1
  c_to_ri19/1 -> Negate5/1
  Negate5/1 -> ri_to_c27/1
  c_to_ri18/2 -> Negate4/1
  Negate4/1 -> ri_to_c26/2
  c_to_ri19/2 -> Negate6/1
  Negate6/1 -> ri_to_c27/2
  fft1/7(out05) -> fft_unscrambler_d88/1
  c_to_ri20/1 -> Negate7/1
  Negate7/1 -> ri_to_c28/1
  c_to_ri20/2 -> Negate8/1
  Negate8/1 -> ri_to_c28/2
  fft1/9(out07) -> fft_unscrambler_d90/1
  fft1/2(out00) -> fft_unscrambler_d83/1
  fft/2(out00) -> fft_unscrambler_d75/1
  ri_to_c1/1 -> fft_unscrambler_d72/1
  fft/3(out01) -> fft_unscrambler_d76/1
  fft1/4(out02) -> fft_unscrambler_d85/1
  fft/4(out02) -> fft_unscrambler_d77/1
  fft/5(out03) -> fft_unscrambler_d78/1
  fft1/6(out04) -> fft_unscrambler_d87/1
  fft/6(out04) -> fft_unscrambler_d79/1
  ri_to_c27/1 -> fft_unscrambler_d97/1
  fft/7(out05) -> fft_unscrambler_d80/1
  fft1/8(out06) -> fft_unscrambler_d89/1
  fft/8(out06) -> fft_unscrambler_d81/1
  fft/9(out07) -> fft_unscrambler_d82/1
  pfb_fir_d56/1 -> Mux/3, pfb_fir/1(sync)
  pfb_fir_d57/1 -> Mux1/3, pfb_fir/2(pol1_in1)
  pfb_fir58/1 -> Mux2/3, pfb_fir/3(pol1_in2)
  pfb_fir_d59/1 -> Mux3/3, pfb_fir/4(pol1_in3)
  pfb_fir_d60/1 -> Mux4/3, pfb_fir/5(pol1_in4)
  pfb_fir_d61/1 -> Mux5/3, pfb_fir/6(pol1_in5)
  pfb_fir_d62/1 -> Mux6/3, pfb_fir/7(pol1_in6)
  pfb_fir_d63/1 -> Mux7/3, pfb_fir/8(pol1_in7)
  pfb_fir_d64/1 -> Mux8/3, pfb_fir/9(pol1_in8)
  fft_unscrambler_d74/1 -> fft_unscrambler_fft_rdy/1(sync_in)
  fft_unscrambler_d75/1 -> fft_unscrambler_d69/1
  fft_unscrambler_d76/1 -> fft_unscrambler_d71/1
  fft_unscrambler_d78/1 -> fft_unscrambler_d92/1
  fft_unscrambler_d79/1 -> fft_unscrambler_d94/1
  fft_unscrambler_d80/1 -> fft_unscrambler_d96/1
  fft_unscrambler_d81/1 -> fft_unscrambler_d98/1
  fft_unscrambler_d82/1 -> fft_unscrambler_d100/1
  fft_unscrambler_d83/1 -> fft_unscrambler_d70/1
  fft_unscrambler_d84/1 -> c_to_ri1/1
  fft_unscrambler_d85/1 -> fft_unscrambler_d91/1
  fft_unscrambler_d86/1 -> c_to_ri18/1
  fft_unscrambler_d88/1 -> c_to_ri19/1
  fft_unscrambler_d89/1 -> fft_unscrambler_d99/1
  fft_unscrambler_d90/1 -> c_to_ri20/1
  fft_unscrambler_d5/1 -> fft_unscrambler_d21/1
  fft_unscrambler_d8/1 -> fft_unscrambler_d117/1
  fft_unscrambler_or1/1 -> overflow/1
  fft_unscrambler_ctr/1 -> bin_ctr/1
  Mux/1 -> pfb_fir_d5/1
  pfb_fir/1(sync_out) -> Mux/2
  Mux1/1 -> pfb_fir_d0/1
  pfb_fir/2(pol1_out1) -> Mux1/2
  Mux2/1 -> pfb_fir_d39/1
  pfb_fir/3(pol1_out2) -> Mux2/2
  Mux3/1 -> pfb_fir_d38/1
  pfb_fir/4(pol1_out3) -> Mux3/2
  Mux4/1 -> pfb_fir_d42/1
  pfb_fir/5(pol1_out4) -> Mux4/2
  Mux5/1 -> pfb_fir_d43/1
  pfb_fir/6(pol1_out5) -> Mux5/2
  pfb_fir/7(pol1_out6) -> Mux6/2
  Mux6/1 -> pfb_fir_d44/1
  Mux7/1 -> pfb_fir_d45/1
  Mux8/1 -> pfb_fir_d46/1
  pfb_fir/9(pol1_out8) -> Mux8/2
  pfb_fir_d51/1 -> Mux9/3, pfb_fir1/1(sync)
  pfb_fir_d52/1 -> Mux10/3, pfb_fir1/2(pol1_in1)
  pfb_fir_d53/1 -> Mux11/3, pfb_fir1/3(pol1_in2)
  pfb_fir_d54/1 -> Mux12/3, pfb_fir1/4(pol1_in3)
  pfb_fir_d55/1 -> Mux13/3, pfb_fir1/5(pol1_in4)
  pfb_fir_d65/1 -> Mux14/3, pfb_fir1/6(pol1_in5)
  pfb_fir_d66/1 -> Mux15/3, pfb_fir1/7(pol1_in6)
  pfb_fir_d67/1 -> Mux16/3, pfb_fir1/8(pol1_in7)
  pfb_fir_d68/1 -> Mux17/3, pfb_fir1/9(pol1_in8)
  Mux9/1 -> pfb_fir_d25/1
  pfb_fir1/1(sync_out) -> Mux9/2
  skip_pfb/1 -> Mux8/1, Mux9/1, Mux17/1, Mux16/1, Mux15/1, Mux14/1, Mux13/1, Mux12/1, Mux11/1, Mux10/1, Mux7/1, Mux6/1, Mux5/1, Mux4/1, Mux3/1, Mux2/1, Mux1/1, Mux/1
  Mux10/1 -> pfb_fir_d24/1
  pfb_fir1/2(pol1_out1) -> Mux10/2
  Mux11/1 -> pfb_fir_d23/1
  pfb_fir1/3(pol1_out2) -> Mux11/2
  Mux12/1 -> pfb_fir_d22/1
  pfb_fir1/4(pol1_out3) -> Mux12/2
  Mux13/1 -> pfb_fir_d26/1
  pfb_fir1/5(pol1_out4) -> Mux13/2
  Mux14/1 -> pfb_fir_d47/1
  pfb_fir1/6(pol1_out5) -> Mux14/2
  pfb_fir1/7(pol1_out6) -> Mux15/2
  Mux15/1 -> pfb_fir_d48/1
  Mux16/1 -> pfb_fir_d49/1
  pfb_fir1/8(pol1_out7) -> Mux16/2
  Mux17/1 -> pfb_fir_d50/1
  pfb_fir1/9(pol1_out8) -> Mux17/2
  ri_to_c28/1 -> fft_unscrambler_d101/1
  ri_to_c26/1 -> fft_unscrambler_d93/1
  fft_unscrambler_d77/1 -> fft_unscrambler_d73/1
  fft_unscrambler_d20/1 -> bin0_t0/1
  fft_unscrambler_d69/1 -> fft_unscrambler_d20/1
  fft_unscrambler_d70/1 -> fft_unscrambler_d102/1
  fft_unscrambler_d71/1 -> fft_unscrambler_d103/1
  fft_unscrambler_d72/1 -> fft_unscrambler_d104/1
  fft_unscrambler_d73/1 -> fft_unscrambler_d105/1
  fft_unscrambler_d91/1 -> fft_unscrambler_d106/1
  fft_unscrambler_d92/1 -> fft_unscrambler_d107/1
  fft_unscrambler_d93/1 -> fft_unscrambler_d108/1
  fft_unscrambler_d94/1 -> fft_unscrambler_d109/1
  fft_unscrambler_d95/1 -> fft_unscrambler_d110/1
  fft_unscrambler_d96/1 -> fft_unscrambler_d111/1
  fft_unscrambler_d97/1 -> fft_unscrambler_d112/1
  fft_unscrambler_d98/1 -> fft_unscrambler_d113/1
  fft_unscrambler_d99/1 -> fft_unscrambler_d114/1
  fft_unscrambler_d100/1 -> fft_unscrambler_d115/1
  fft_unscrambler_d101/1 -> fft_unscrambler_d116/1
  fft_unscrambler_d102/1 -> bin0_t1/1
  fft_unscrambler_d103/1 -> bin1_t0/1
  fft_unscrambler_d104/1 -> bin1_t1/1
  fft_unscrambler_d105/1 -> bin2_t0/1
  fft_unscrambler_d106/1 -> bin2_t1/1
  fft_unscrambler_d107/1 -> bin3_t0/1
  fft_unscrambler_d108/1 -> bin3_t1/1
  fft_unscrambler_d109/1 -> bin4_t0/1
  fft_unscrambler_d110/1 -> bin4_t1/1
  fft_unscrambler_d111/1 -> bin5_t0/1
  fft_unscrambler_d112/1 -> bin5_t1/1
  fft_unscrambler_d113/1 -> bin6_t0/1
  fft_unscrambler_d114/1 -> bin6_t1/1
  fft_unscrambler_d115/1 -> bin7_t0/1
  fft_unscrambler_d116/1 -> bin7_t1/1
  fft_unscrambler_d21/1 -> fft_unscrambler_or1/1
  fft_unscrambler_d117/1 -> fft_unscrambler_or1/2
  pfb_fir/8(pol1_out7) -> Mux7/2
  pfb_fir_d4/1 -> fft_stage_1_d10/1
  pfb_fir_d6/1 -> fft_stage_1_d9/1

== system dark_pfb_core10/PFB/delay_bram1 ==
  din : Inport
  constant : xbsIndex_r4/Constant  const=1  gui_display_data_type=Fixed-point  arith_type=Boolean  n_bits=16  bin_pt=14  preci_type=Single  exp_width=8  frac_width=24  explicit_period=on  period=1  equ=P=C  opselect=C  inp2=PCIN>>17  opr=+  inp1=P  carry=CIN  dbl_ovrd=off  block_type=constant
  counter : xbsIndex_r4/Counter  cnt_type=Count Limited  cnt_to=DelayLen - bram_latency - 1  operation=Up  start_count=0  cnt_by_val=1  arith_type=Unsigned  n_bits=7  bin_pt=0  load_pin=off  rst=off  en=off  explicit_period=on  period=1  dbl_ovrd=off  use_behavioral_HDL=off  implementation=DSP48  use_rpm=on  block_type=counter
  ram : xbsIndex_r4/Single Port RAM  depth=128  initVector=0  distributed_mem=Block RAM  write_mode=Read Before Write  rst=off  init_reg=0  en=off  latency=bram_latency  dbl_ovrd=off  optimize=Area  block_type=spram
  dout : Outport
  wires:
  counter/1 -> ram/1
  din/1 -> ram/2
  constant/1 -> ram/3
  ram/1 -> dout/1

== system dark_pfb_core10/PFB/delay_bram2 ==
  din : Inport
  constant : xbsIndex_r4/Constant  const=1  gui_display_data_type=Fixed-point  arith_type=Boolean  n_bits=16  bin_pt=14  preci_type=Single  exp_width=8  frac_width=24  explicit_period=on  period=1  equ=P=C  opselect=C  inp2=PCIN>>17  opr=+  inp1=P  carry=CIN  dbl_ovrd=off  block_type=constant
  counter : xbsIndex_r4/Counter  cnt_type=Count Limited  cnt_to=DelayLen - bram_latency - 1  operation=Up  start_count=0  cnt_by_val=1  arith_type=Unsigned  n_bits=7  bin_pt=0  load_pin=off  rst=off  en=off  explicit_period=on  period=1  dbl_ovrd=off  use_behavioral_HDL=off  implementation=DSP48  use_rpm=on  block_type=counter
  ram : xbsIndex_r4/Single Port RAM  depth=128  initVector=0  distributed_mem=Block RAM  write_mode=Read Before Write  rst=off  init_reg=0  en=off  latency=bram_latency  dbl_ovrd=off  optimize=Area  block_type=spram
  dout : Outport
  wires:
  ram/1 -> dout/1
  constant/1 -> ram/3
  din/1 -> ram/2
  counter/1 -> ram/1

== system dark_pfb_core10/PFB/delay_bram3 ==
  din : Inport
  constant : xbsIndex_r4/Constant  const=1  gui_display_data_type=Fixed-point  arith_type=Boolean  n_bits=16  bin_pt=14  preci_type=Single  exp_width=8  frac_width=24  explicit_period=on  period=1  equ=P=C  opselect=C  inp2=PCIN>>17  opr=+  inp1=P  carry=CIN  dbl_ovrd=off  block_type=constant
  counter : xbsIndex_r4/Counter  cnt_type=Count Limited  cnt_to=DelayLen - bram_latency - 1  operation=Up  start_count=0  cnt_by_val=1  arith_type=Unsigned  n_bits=7  bin_pt=0  load_pin=off  rst=off  en=off  explicit_period=on  period=1  dbl_ovrd=off  use_behavioral_HDL=off  implementation=DSP48  use_rpm=on  block_type=counter
  ram : xbsIndex_r4/Single Port RAM  depth=128  initVector=0  distributed_mem=Block RAM  write_mode=Read Before Write  rst=off  init_reg=0  en=off  latency=bram_latency  dbl_ovrd=off  optimize=Area  block_type=spram
  dout : Outport
  wires:
  counter/1 -> ram/1
  din/1 -> ram/2
  constant/1 -> ram/3
  ram/1 -> dout/1

== system dark_pfb_core10/PFB/delay_bram4 ==
  din : Inport
  constant : xbsIndex_r4/Constant  const=1  gui_display_data_type=Fixed-point  arith_type=Boolean  n_bits=16  bin_pt=14  preci_type=Single  exp_width=8  frac_width=24  explicit_period=on  period=1  equ=P=C  opselect=C  inp2=PCIN>>17  opr=+  inp1=P  carry=CIN  dbl_ovrd=off  block_type=constant
  counter : xbsIndex_r4/Counter  cnt_type=Count Limited  cnt_to=DelayLen - bram_latency - 1  operation=Up  start_count=0  cnt_by_val=1  arith_type=Unsigned  n_bits=7  bin_pt=0  load_pin=off  rst=off  en=off  explicit_period=on  period=1  dbl_ovrd=off  use_behavioral_HDL=off  implementation=DSP48  use_rpm=on  block_type=counter
  ram : xbsIndex_r4/Single Port RAM  depth=128  initVector=0  distributed_mem=Block RAM  write_mode=Read Before Write  rst=off  init_reg=0  en=off  latency=bram_latency  dbl_ovrd=off  optimize=Area  block_type=spram
  dout : Outport
  wires:
  ram/1 -> dout/1
  constant/1 -> ram/3
  din/1 -> ram/2
  counter/1 -> ram/1

== system dark_pfb_core10/PFB/delay_bram5 ==
  din : Inport
  constant : xbsIndex_r4/Constant  const=1  gui_display_data_type=Fixed-point  arith_type=Boolean  n_bits=16  bin_pt=14  preci_type=Single  exp_width=8  frac_width=24  explicit_period=on  period=1  equ=P=C  opselect=C  inp2=PCIN>>17  opr=+  inp1=P  carry=CIN  dbl_ovrd=off  block_type=constant
  counter : xbsIndex_r4/Counter  cnt_type=Count Limited  cnt_to=DelayLen - bram_latency - 1  operation=Up  start_count=0  cnt_by_val=1  arith_type=Unsigned  n_bits=7  bin_pt=0  load_pin=off  rst=off  en=off  explicit_period=on  period=1  dbl_ovrd=off  use_behavioral_HDL=off  implementation=DSP48  use_rpm=on  block_type=counter
  ram : xbsIndex_r4/Single Port RAM  depth=128  initVector=0  distributed_mem=Block RAM  write_mode=Read Before Write  rst=off  init_reg=0  en=off  latency=bram_latency  dbl_ovrd=off  optimize=Area  block_type=spram
  dout : Outport
  wires:
  counter/1 -> ram/1
  din/1 -> ram/2
  constant/1 -> ram/3
  ram/1 -> dout/1

== system dark_pfb_core10/PFB/delay_bram6 ==
  din : Inport
  constant : xbsIndex_r4/Constant  const=1  gui_display_data_type=Fixed-point  arith_type=Boolean  n_bits=16  bin_pt=14  preci_type=Single  exp_width=8  frac_width=24  explicit_period=on  period=1  equ=P=C  opselect=C  inp2=PCIN>>17  opr=+  inp1=P  carry=CIN  dbl_ovrd=off  block_type=constant
  counter : xbsIndex_r4/Counter  cnt_type=Count Limited  cnt_to=DelayLen - bram_latency - 1  operation=Up  start_count=0  cnt_by_val=1  arith_type=Unsigned  n_bits=7  bin_pt=0  load_pin=off  rst=off  en=off  explicit_period=on  period=1  dbl_ovrd=off  use_behavioral_HDL=off  implementation=DSP48  use_rpm=on  block_type=counter
  ram : xbsIndex_r4/Single Port RAM  depth=128  initVector=0  distributed_mem=Block RAM  write_mode=Read Before Write  rst=off  init_reg=0  en=off  latency=bram_latency  dbl_ovrd=off  optimize=Area  block_type=spram
  dout : Outport
  wires:
  ram/1 -> dout/1
  constant/1 -> ram/3
  din/1 -> ram/2
  counter/1 -> ram/1
